simulink library "Garbage"  (R2023a)
settings: Solver=ode45  StartTime=0.0  StopTime=10.0  RelTol=1e-3  EnableMultiTasking=on
undefined symbols (not defined in the file or in its folder): c, ending_column_index, ending_row_index, framebuffer_16x1, framebuffer_2x2, framebuffer_4x4, framebuffer_8x8, HEIGHT, hot, POS_X, POS_Y, starting_column_index, starting_row_index, WIDTH
libraries: Memory_Blocks_Lib x459, simulink x235, simulink_extras x132, dspmtrx3 x116, Bit_Multiplexers_Lib x67, Bit_Demultiplexers_Lib x49, Byte_Multiplexers_Lib x40, Miscellaneous x38, Computer_Library x17, Decoders_Lib x14, commutil2 x12, Arithmetic_Operations_Lib x8
(part 14 of 26)

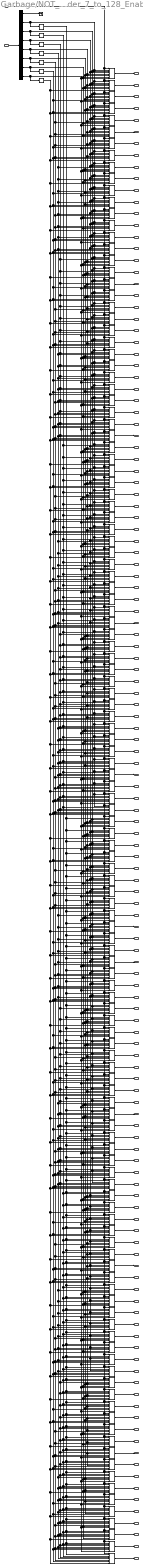
== system Garbage/NOT_USED_Decoder_7_to_128_Enable ==
  Enable(1b) : Inport
  Addr(1B) : Inport  Port=2
  Lo`gical Operator{1,3,5..6} (4 alike) : Logic  Operator=NOT  IconShape=distinctive  AllPortsSameDT=off  OutDataTypeStr=boolean
  Logical Operator{1..4,6..21,24..81,84..111,113..134} (128 alike) : Logic  Inputs=8  IconShape=distinctive  AllPortsSameDT=off  OutDataTypeStr=boolean
  Logical Operator112 : Logic  Operator=NOT  IconShape=distinctive  AllPortsSameDT=off  OutDataTypeStr=boolean
  Logical Operator82 : Logic  Operator=NOT  IconShape=distinctive  AllPortsSameDT=off  OutDataTypeStr=boolean
  Logical Operator83 : Logic  Operator=NOT  IconShape=distinctive  AllPortsSameDT=off  OutDataTypeStr=boolean
  Terminator : Terminator
  xyz1 : BusSelector  OutputSignals=signal1,signal2,signal3,signal4,signal5,signal6,signal7,signal8
  Select_127(1b) : Outport
  Select_126(1b) : Outport  Port=2
  Select_125(1b) : Outport  Port=3
  Select_124(1b) : Outport  Port=4
  Select_123(1b) : Outport  Port=5
  Select_122(1b) : Outport  Port=6
  Select_121(1b) : Outport  Port=7
  Select_120(1b) : Outport  Port=8
  Select_119(1b) : Outport  Port=9
  Select_118(1b) : Outport  Port=10
  Select_117(1b) : Outport  Port=11
  Select_116(1b) : Outport  Port=12
  Select_115(1b) : Outport  Port=13
  Select_114(1b) : Outport  Port=14
  Select_113(1b) : Outport  Port=15
  Select_112(1b) : Outport  Port=16
  Select_111(1b) : Outport  Port=17
  Select_110(1b) : Outport  Port=18
  Select_109(1b) : Outport  Port=19
  Select_108(1b) : Outport  Port=20
  Select_107(1b) : Outport  Port=21
  Select_106(1b) : Outport  Port=22
  Select_105(1b) : Outport  Port=23
  Select_104(1b) : Outport  Port=24
  Select_103(1b) : Outport  Port=25
  Select_102(1b) : Outport  Port=26
  Select_101(1b) : Outport  Port=27
  Select_100(1b) : Outport  Port=28
  Select_099(1b) : Outport  Port=29
  Select_098(1b) : Outport  Port=30
  Select_097(1b) : Outport  Port=31
  Select_096(1b) : Outport  Port=32
  Select_095(1b) : Outport  Port=33
  Select_094(1b) : Outport  Port=34
  Select_093(1b) : Outport  Port=35
  Select_092(1b) : Outport  Port=36
  Select_091(1b) : Outport  Port=37
  Select_090(1b) : Outport  Port=38
  Select_089(1b) : Outport  Port=39
  Select_088(1b) : Outport  Port=40
  Select_087(1b) : Outport  Port=41
  Select_086(1b) : Outport  Port=42
  Select_085(1b) : Outport  Port=43
  Select_084(1b) : Outport  Port=44
  Select_083(1b) : Outport  Port=45
  Select_082(1b) : Outport  Port=46
  Select_081(1b) : Outport  Port=47
  Select_080(1b) : Outport  Port=48
  Select_079(1b) : Outport  Port=49
  Select_078(1b) : Outport  Port=50
  Select_077(1b) : Outport  Port=51
  Select_076(1b) : Outport  Port=52
  Select_075(1b) : Outport  Port=53
  Select_074(1b) : Outport  Port=54
  Select_073(1b) : Outport  Port=55
  Select_072(1b) : Outport  Port=56
  Select_071(1b) : Outport  Port=57
  Select_070(1b) : Outport  Port=58
  Select_069(1b) : Outport  Port=59
  Select_068(1b) : Outport  Port=60
  Select_067(1b) : Outport  Port=61
  Select_066(1b) : Outport  Port=62
  Select_065(1b) : Outport  Port=63
  Select_064(1b) : Outport  Port=64
  Select_063(1b) : Outport  Port=65
  Select_062(1b) : Outport  Port=66
  Select_061(1b) : Outport  Port=67
  Select_060(1b) : Outport  Port=68
  Select_059(1b) : Outport  Port=69
  Select_058(1b) : Outport  Port=70
  Select_057(1b) : Outport  Port=71
  Select_056(1b) : Outport  Port=72
  Select_055(1b) : Outport  Port=73
  Select_054(1b) : Outport  Port=74
  Select_053(1b) : Outport  Port=75
  Select_052(1b) : Outport  Port=76
  Select_051(1b) : Outport  Port=77
  Select_050(1b) : Outport  Port=78
  Select_049(1b) : Outport  Port=79
  Select_048(1b) : Outport  Port=80
  Select_047(1b) : Outport  Port=81
  Select_046(1b) : Outport  Port=82
  Select_045(1b) : Outport  Port=83
  Select_044(1b) : Outport  Port=84
  Select_043(1b) : Outport  Port=85
  Select_042(1b) : Outport  Port=86
  Select_041(1b) : Outport  Port=87
  Select_040(1b) : Outport  Port=88
  Select_039(1b) : Outport  Port=89
  Select_038(1b) : Outport  Port=90
  Select_037(1b) : Outport  Port=91
  Select_036(1b) : Outport  Port=92
  Select_035(1b) : Outport  Port=93
  Select_034(1b) : Outport  Port=94
  Select_033(1b) : Outport  Port=95
  Select_032(1b) : Outport  Port=96
  Select_031(1b) : Outport  Port=97
  Select_030(1b) : Outport  Port=98
  Select_029(1b) : Outport  Port=99
  Select_028(1b) : Outport  Port=100
  Select_027(1b) : Outport  Port=101
  Select_026(1b) : Outport  Port=102
  Select_025(1b) : Outport  Port=103
  Select_024(1b) : Outport  Port=104
  Select_023(1b) : Outport  Port=105
  Select_022(1b) : Outport  Port=106
  Select_021(1b) : Outport  Port=107
  Select_020(1b) : Outport  Port=108
  Select_019(1b) : Outport  Port=109
  Select_018(1b) : Outport  Port=110
  Select_017(1b) : Outport  Port=111
  Select_016(1b) : Outport  Port=112
  Select_015(1b) : Outport  Port=113
  Select_014(1b) : Outport  Port=114
  Select_013(1b) : Outport  Port=115
  Select_012(1b) : Outport  Port=116
  Select_011(1b) : Outport  Port=117
  Select_010(1b) : Outport  Port=118
  Select_009(1b) : Outport  Port=119
  Select_008(1b) : Outport  Port=120
  Select_007(1b) : Outport  Port=121
  Select_006(1b) : Outport  Port=122
  Select_005(1b) : Outport  Port=123
  Select_004(1b) : Outport  Port=124
  Select_003(1b) : Outport  Port=125
  Select_002(1b) : Outport  Port=126
  Select_001(1b) : Outport  Port=127
  Select_000(1b) : Outport  Port=128
  wires:
  Logical Operator16/1 -> Select_048(1b)/1
  Logical Operator15/1 -> Select_049(1b)/1
  Logical Operator14/1 -> Select_050(1b)/1
  Logical Operator13/1 -> Select_051(1b)/1
  Logical Operator12/1 -> Select_052(1b)/1
  Logical Operator11/1 -> Select_053(1b)/1
  Logical Operator10/1 -> Select_054(1b)/1
  Logical Operator9/1 -> Select_055(1b)/1
  Logical Operator8/1 -> Select_056(1b)/1
  Logical Operator7/1 -> Select_057(1b)/1
  Logical Operator6/1 -> Select_058(1b)/1
  Logical Operator4/1 -> Select_059(1b)/1
  Logical Operator1/1 -> Select_062(1b)/1
  Logical Operator2/1 -> Select_061(1b)/1
  Logical Operator3/1 -> Select_060(1b)/1
  Logical Operator63/1 -> Select_063(1b)/1
  Logical Operator26/1 -> Select_032(1b)/1
  Logical Operator25/1 -> Select_033(1b)/1
  Logical Operator24/1 -> Select_034(1b)/1
  Logical Operator21/1 -> Select_035(1b)/1
  Logical Operator20/1 -> Select_036(1b)/1
  Logical Operator19/1 -> Select_037(1b)/1
  Logical Operator18/1 -> Select_038(1b)/1
  Logical Operator34/1 -> Select_039(1b)/1
  Logical Operator33/1 -> Select_040(1b)/1
  Logical Operator32/1 -> Select_041(1b)/1
  Logical Operator30/1 -> Select_042(1b)/1
  Logical Operator29/1 -> Select_043(1b)/1
  Logical Operator17/1 -> Select_046(1b)/1
  Logical Operator27/1 -> Select_045(1b)/1
  Logical Operator28/1 -> Select_044(1b)/1
  Logical Operator31/1 -> Select_047(1b)/1
  xyz1/3 -> Logical Operator76/3, Logical Operator17/3, Logical Operator26/3, Logical Operator25/3, Logical Operator24/3, Logical Operator21/3, Logical Operator20/3, Logical Operator19/3, Logical Operator18/3, Logical Operator34/3, Logical Operator33/3, Logical Operator32/3, Logical Operator30/3, Logical Operator29/3, Logical Operator28/3, Logical Operator27/3, Logical Operator31/3, Logical Operator16/3, Logical Operator15/3, Logical Operator14/3, Logical Operator13/3, Logical Operator12/3, Logical Operator11/3, Logical Operator10/3, Logical Operator9/3, Logical Operator8/3, Logical Operator7/3, Logical Operator6/3, Logical Operator4/3, Logical Operator3/3, Logical Operator2/3, Logical Operator1/3, Logical Operator63/3, Logical Operator86/3, Logical Operator85/3, Logical Operator84/3, Logical Operator81/3, Logical Operator80/3, Logical Operator78/3, Logical Operator77/3, Logical Operator95/3, Logical Operator94/3, Logical Operator93/3, Logical Operator91/3, Logical Operator89/3, Logical Operator88/3, Logical Operator87/3, Logical Operator92/3, Logical Operator75/3, Logical Operator74/3, Logical Operator73/3, Logical Operator72/3, Logical Operator71/3, Logical Operator70/3, Logical Operator69/3, Logical Operator134/3, Logical Operator133/3, Logical Operator132/3, Logical Operator123/3, Logical Operator101/3, Logical Operator90/3, Logical Operator79/3, Logical Operator68/3, Logical Operator127/3, Lo`gical Operator5/1  "<signal3>"
  Logical Operator42/1 -> Select_016(1b)/1
  Logical Operator41/1 -> Select_017(1b)/1
  Logical Operator40/1 -> Select_018(1b)/1
  Logical Operator39/1 -> Select_019(1b)/1
  Logical Operator38/1 -> Select_020(1b)/1
  Logical Operator37/1 -> Select_021(1b)/1
  Logical Operator36/1 -> Select_022(1b)/1
  Logical Operator50/1 -> Select_023(1b)/1
  Logical Operator49/1 -> Select_024(1b)/1
  Logical Operator48/1 -> Select_025(1b)/1
  Logical Operator46/1 -> Select_026(1b)/1
  Logical Operator45/1 -> Select_027(1b)/1
  Logical Operator35/1 -> Select_030(1b)/1
  Logical Operator43/1 -> Select_029(1b)/1
  Logical Operator44/1 -> Select_028(1b)/1
  Logical Operator47/1 -> Select_031(1b)/1
  Logical Operator82/1 -> Logical Operator93/7, Logical Operator105/7, Logical Operator106/7, Logical Operator122/7, Logical Operator124/7, Logical Operator32/7, Logical Operator43/7, Logical Operator44/7, Logical Operator59/7, Logical Operator60/7, Logical Operator58/7, Logical Operator57/7, Logical Operator54/7, Logical Operator53/7, Logical Operator66/7, Logical Operator65/7, Logical Operator42/7, Logical Operator41/7, Logical Operator38/7, Logical Operator37/7, Logical Operator49/7, Logical Operator48/7, Logical Operator26/7, Logical Operator25/7, Logical Operator20/7, Logical Operator19/7, Logical Operator33/7, Logical Operator28/7, Logical Operator27/7, Logical Operator16/7, Logical Operator15/7, Logical Operator12/7, Logical Operator11/7, Logical Operator8/7, Logical Operator7/7, Logical Operator3/7, Logical Operator2/7, Logical Operator121/7, Logical Operator120/7, Logical Operator117/7, Logical Operator116/7, Logical Operator130/7, Logical Operator129/7, Logical Operator104/7, Logical Operator103/7, Logical Operator99/7, Logical Operator98/7, Logical Operator111/7, Logical Operator110/7, Logical Operator86/7, Logical Operator85/7, Logical Operator80/7, Logical Operator78/7, Logical Operator94/7, Logical Operator88/7, Logical Operator87/7, Logical Operator75/7, Logical Operator74/7, Logical Operator71/7, Logical Operator70/7, Logical Operator133/7, Logical Operator132/7, Logical Operator90/7, Logical Operator79/7
  xyz1/8 -> Logical Operator83/1, Logical Operator127/8, Logical Operator63/8, Logical Operator57/8, Logical Operator55/8, Logical Operator53/8, Logical Operator67/8, Logical Operator65/8, Logical Operator61/8, Logical Operator59/8, Logical Operator64/8, Logical Operator41/8, Logical Operator39/8, Logical Operator37/8, Logical Operator50/8, Logical Operator48/8, Logical Operator45/8, Logical Operator43/8, Logical Operator47/8, Logical Operator25/8, Logical Operator21/8, Logical Operator19/8, Logical Operator34/8, Logical Operator32/8, Logical Operator29/8, Logical Operator27/8, Logical Operator31/8, Logical Operator15/8, Logical Operator13/8, Logical Operator11/8, Logical Operator9/8, Logical Operator7/8, Logical Operator4/8, Logical Operator2/8, Logical Operator120/8, Logical Operator118/8, Logical Operator116/8, Logical Operator131/8, Logical Operator129/8, Logical Operator125/8, Logical Operator122/8, Logical Operator128/8, Logical Operator103/8, Logical Operator100/8, Logical Operator98/8, Logical Operator113/8, Logical Operator110/8, Logical Operator107/8, Logical Operator105/8, Logical Operator109/8, Logical Operator85/8, Logical Operator81/8, Logical Operator78/8, Logical Operator95/8, Logical Operator93/8, Logical Operator89/8, Logical Operator87/8, Logical Operator92/8, Logical Operator74/8, Logical Operator72/8, Logical Operator70/8, Logical Operator134/8, Logical Operator132/8, Logical Operator101/8, Logical Operator79/8  "<signal8>"
  Logical Operator83/1 -> Logical Operator86/8, Logical Operator104/8, Logical Operator26/8, Logical Operator42/8, Logical Operator58/8, Logical Operator56/8, Logical Operator54/8, Logical Operator52/8, Logical Operator66/8, Logical Operator62/8, Logical Operator60/8, Logical Operator51/8, Logical Operator40/8, Logical Operator38/8, Logical Operator36/8, Logical Operator49/8, Logical Operator46/8, Logical Operator44/8, Logical Operator35/8, Logical Operator24/8, Logical Operator20/8, Logical Operator18/8, Logical Operator33/8, Logical Operator30/8, Logical Operator28/8, Logical Operator17/8, Logical Operator16/8, Logical Operator14/8, Logical Operator12/8, Logical Operator10/8, Logical Operator8/8, Logical Operator6/8, Logical Operator3/8, Logical Operator1/8, Logical Operator121/8, Logical Operator119/8, Logical Operator117/8, Logical Operator115/8, Logical Operator130/8, Logical Operator126/8, Logical Operator124/8, Logical Operator114/8, Logical Operator102/8, Logical Operator99/8, Logical Operator97/8, Logical Operator111/8, Logical Operator108/8, Logical Operator106/8, Logical Operator96/8, Logical Operator84/8, Logical Operator80/8, Logical Operator77/8, Logical Operator94/8, Logical Operator91/8, Logical Operator88/8, Logical Operator76/8, Logical Operator75/8, Logical Operator73/8, Logical Operator71/8, Logical Operator69/8, Logical Operator133/8, Logical Operator123/8, Logical Operator90/8, Logical Operator68/8
  Logical Operator112/1 -> Logical Operator86/6, Logical Operator26/6, Logical Operator58/6, Logical Operator57/6, Logical Operator56/6, Logical Operator55/6, Logical Operator66/6, Logical Operator65/6, Logical Operator62/6, Logical Operator61/6, Logical Operator42/6, Logical Operator41/6, Logical Operator40/6, Logical Operator39/6, Logical Operator49/6, Logical Operator48/6, Logical Operator46/6, Logical Operator45/6, Logical Operator25/6, Logical Operator24/6, Logical Operator21/6, Logical Operator33/6, Logical Operator32/6, Logical Operator30/6, Logical Operator29/6, Logical Operator16/6, Logical Operator15/6, Logical Operator14/6, Logical Operator13/6, Logical Operator8/6, Logical Operator7/6, Logical Operator6/6, Logical Operator4/6, Logical Operator121/6, Logical Operator120/6, Logical Operator119/6, Logical Operator118/6, Logical Operator130/6, Logical Operator129/6, Logical Operator126/6, Logical Operator125/6, Logical Operator104/6, Logical Operator103/6, Logical Operator102/6, Logical Operator100/6, Logical Operator111/6, Logical Operator110/6, Logical Operator108/6, Logical Operator107/6, Logical Operator85/6, Logical Operator84/6, Logical Operator81/6, Logical Operator94/6, Logical Operator93/6, Logical Operator91/6, Logical Operator89/6, Logical Operator75/6, Logical Operator74/6, Logical Operator73/6, Logical Operator72/6, Logical Operator133/6, Logical Operator132/6, Logical Operator123/6, Logical Operator101/6
  xyz1/5 -> Lo`gical Operator6/1, Logical Operator127/5, Logical Operator68/5, Logical Operator79/5, Logical Operator90/5, Logical Operator101/5, Logical Operator123/5, Logical Operator132/5, Logical Operator133/5, Logical Operator92/5, Logical Operator76/5, Logical Operator87/5, Logical Operator88/5, Logical Operator89/5, Logical Operator91/5, Logical Operator94/5, Logical Operator109/5, Logical Operator96/5, Logical Operator105/5, Logical Operator106/5, Logical Operator107/5, Logical Operator108/5, Logical Operator110/5, Logical Operator111/5, Logical Operator128/5, Logical Operator114/5, Logical Operator122/5, Logical Operator124/5, Logical Operator125/5, Logical Operator126/5, Logical Operator63/5, Logical Operator1/5, Logical Operator2/5, Logical Operator3/5, Logical Operator4/5, Logical Operator6/5, Logical Operator7/5, Logical Operator8/5, Logical Operator31/5, Logical Operator17/5, Logical Operator27/5, Logical Operator28/5, Logical Operator29/5, Logical Operator30/5, Logical Operator33/5, Logical Operator47/5, Logical Operator35/5, Logical Operator43/5, Logical Operator44/5, Logical Operator45/5, Logical Operator46/5, Logical Operator48/5, Logical Operator49/5, Logical Operator64/5, Logical Operator51/5, Logical Operator59/5, Logical Operator60/5, Logical Operator61/5, Logical Operator62/5, Logical Operator66/5, Logical Operator65/5, Logical Operator32/5, Logical Operator130/5, Logical Operator129/5, Logical Operator93/5  "<signal5>"
  xyz1/7 -> Logical Operator82/1, Logical Operator127/7, Logical Operator91/7, Logical Operator95/7, Logical Operator84/7, Logical Operator109/7, Logical Operator119/7, Logical Operator63/7, Logical Operator30/7, Logical Operator34/7, Logical Operator24/7, Logical Operator47/7, Logical Operator56/7, Logical Operator55/7, Logical Operator52/7, Logical Operator67/7, Logical Operator62/7, Logical Operator61/7, Logical Operator51/7, Logical Operator64/7, Logical Operator40/7, Logical Operator39/7, Logical Operator36/7, Logical Operator50/7, Logical Operator46/7, Logical Operator45/7, Logical Operator35/7, Logical Operator21/7, Logical Operator18/7, Logical Operator29/7, Logical Operator17/7, Logical Operator31/7, Logical Operator14/7, Logical Operator13/7, Logical Operator10/7, Logical Operator9/7, Logical Operator6/7, Logical Operator4/7, Logical Operator1/7, Logical Operator118/7, Logical Operator115/7, Logical Operator131/7, Logical Operator126/7, Logical Operator125/7, Logical Operator114/7, Logical Operator128/7, Logical Operator102/7, Logical Operator100/7, Logical Operator97/7, Logical Operator113/7, Logical Operator108/7, Logical Operator107/7, Logical Operator96/7, Logical Operator81/7, Logical Operator77/7, Logical Operator89/7, Logical Operator76/7, Logical Operator92/7, Logical Operator73/7, Logical Operator72/7, Logical Operator69/7, Logical Operator134/7, Logical Operator123/7, Logical Operator101/7, Logical Operator68/7  "<signal7>"
  xyz1/6 -> Logical Operator112/1, Logical Operator127/6, Logical Operator68/6, Logical Operator134/6, Logical Operator69/6, Logical Operator70/6, Logical Operator71/6, Logical Operator92/6, Logical Operator76/6, Logical Operator95/6, Logical Operator77/6, Logical Operator78/6, Logical Operator80/6, Logical Operator109/6, Logical Operator96/6, Logical Operator113/6, Logical Operator97/6, Logical Operator98/6, Logical Operator99/6, Logical Operator128/6, Logical Operator114/6, Logical Operator131/6, Logical Operator115/6, Logical Operator63/6, Logical Operator1/6, Logical Operator9/6, Logical Operator10/6, Logical Operator11/6, Logical Operator12/6, Logical Operator31/6, Logical Operator17/6, Logical Operator34/6, Logical Operator18/6, Logical Operator19/6, Logical Operator20/6, Logical Operator47/6, Logical Operator35/6, Logical Operator50/6, Logical Operator36/6, Logical Operator37/6, Logical Operator38/6, Logical Operator64/6, Logical Operator51/6, Logical Operator67/6, Logical Operator52/6, Logical Operator54/6, Logical Operator53/6, Logical Operator60/6, Logical Operator59/6, Logical Operator44/6, Logical Operator43/6, Logical Operator28/6, Logical Operator27/6, Logical Operator3/6, Logical Operator2/6, Logical Operator117/6, Logical Operator116/6, Logical Operator124/6, Logical Operator122/6, Logical Operator106/6, Logical Operator105/6, Logical Operator88/6, Logical Operator87/6, Logical Operator90/6, Logical Operator79/6  "<signal6>"
  Lo`gical Operator6/1 -> Logical Operator77/5, Logical Operator78/5, Logical Operator80/5, Logical Operator81/5, Logical Operator84/5, Logical Operator85/5, Logical Operator113/5, Logical Operator97/5, Logical Operator98/5, Logical Operator99/5, Logical Operator100/5, Logical Operator102/5, Logical Operator103/5, Logical Operator131/5, Logical Operator115/5, Logical Operator116/5, Logical Operator117/5, Logical Operator118/5, Logical Operator119/5, Logical Operator18/5, Logical Operator19/5, Logical Operator20/5, Logical Operator21/5, Logical Operator24/5, Logical Operator25/5, Logical Operator50/5, Logical Operator36/5, Logical Operator37/5, Logical Operator38/5, Logical Operator39/5, Logical Operator40/5, Logical Operator41/5, Logical Operator67/5, Logical Operator52/5, Logical Operator53/5, Logical Operator54/5, Logical Operator55/5, Logical Operator56/5, Logical Operator58/5, Logical Operator57/5, Logical Operator42/5, Logical Operator26/5, Logical Operator34/5, Logical Operator16/5, Logical Operator15/5, Logical Operator14/5, Logical Operator13/5, Logical Operator12/5, Logical Operator11/5, Logical Operator10/5, Logical Operator9/5, Logical Operator121/5, Logical Operator120/5, Logical Operator104/5, Logical Operator86/5, Logical Operator95/5, Logical Operator75/5, Logical Operator74/5, Logical Operator73/5, Logical Operator72/5, Logical Operator71/5, Logical Operator70/5, Logical Operator69/5, Logical Operator134/5
  Logical Operator58/1 -> Select_000(1b)/1
  Logical Operator57/1 -> Select_001(1b)/1
  Logical Operator56/1 -> Select_002(1b)/1
  Logical Operator55/1 -> Select_003(1b)/1
  Logical Operator54/1 -> Select_004(1b)/1
  Logical Operator53/1 -> Select_005(1b)/1
  Logical Operator52/1 -> Select_006(1b)/1
  Logical Operator67/1 -> Select_007(1b)/1
  Logical Operator66/1 -> Select_008(1b)/1
  Logical Operator65/1 -> Select_009(1b)/1
  Logical Operator62/1 -> Select_010(1b)/1
  Logical Operator61/1 -> Select_011(1b)/1
  Logical Operator51/1 -> Select_014(1b)/1
  Logical Operator59/1 -> Select_013(1b)/1
  Logical Operator60/1 -> Select_012(1b)/1
  Logical Operator64/1 -> Select_015(1b)/1
  Lo`gical Operator3/1 -> Logical Operator78/4, Logical Operator86/4, Logical Operator122/4, Logical Operator126/4, Logical Operator19/4, Logical Operator26/4, Logical Operator59/4, Logical Operator62/4, Logical Operator58/4, Logical Operator57/4, Logical Operator56/4, Logical Operator55/4, Logical Operator54/4, Logical Operator53/4, Logical Operator52/4, Logical Operator67/4, Logical Operator66/4, Logical Operator65/4, Logical Operator61/4, Logical Operator60/4, Logical Operator51/4, Logical Operator64/4, Logical Operator25/4, Logical Operator24/4, Logical Operator21/4, Logical Operator20/4, Logical Operator18/4, Logical Operator34/4, Logical Operator33/4, Logical Operator32/4, Logical Operator30/4, Logical Operator29/4, Logical Operator28/4, Logical Operator27/4, Logical Operator17/4, Logical Operator31/4, Logical Operator121/4, Logical Operator120/4, Logical Operator119/4, Logical Operator118/4, Logical Operator117/4, Logical Operator116/4, Logical Operator115/4, Logical Operator131/4, Logical Operator130/4, Logical Operator129/4, Logical Operator125/4, Logical Operator124/4, Logical Operator114/4, Logical Operator128/4, Logical Operator85/4, Logical Operator84/4, Logical Operator81/4, Logical Operator80/4, Logical Operator77/4, Logical Operator95/4, Logical Operator94/4, Logical Operator93/4, Logical Operator91/4, Logical Operator89/4, Logical Operator88/4, Logical Operator87/4, Logical Operator76/4, Logical Operator92/4
  xyz1/4 -> Lo`gical Operator3/1, Logical Operator109/4, Logical Operator106/4, Logical Operator107/4, Logical Operator108/4, Logical Operator111/4, Logical Operator113/4, Logical Operator98/4, Logical Operator100/4, Logical Operator47/4, Logical Operator44/4, Logical Operator45/4, Logical Operator46/4, Logical Operator49/4, Logical Operator50/4, Logical Operator37/4, Logical Operator39/4, Logical Operator42/4, Logical Operator41/4, Logical Operator40/4, Logical Operator38/4, Logical Operator36/4, Logical Operator48/4, Logical Operator43/4, Logical Operator35/4, Logical Operator16/4, Logical Operator15/4, Logical Operator14/4, Logical Operator13/4, Logical Operator12/4, Logical Operator11/4, Logical Operator10/4, Logical Operator9/4, Logical Operator8/4, Logical Operator7/4, Logical Operator6/4, Logical Operator4/4, Logical Operator3/4, Logical Operator2/4, Logical Operator1/4, Logical Operator63/4, Logical Operator104/4, Logical Operator103/4, Logical Operator102/4, Logical Operator99/4, Logical Operator97/4, Logical Operator110/4, Logical Operator105/4, Logical Operator96/4, Logical Operator75/4, Logical Operator74/4, Logical Operator73/4, Logical Operator72/4, Logical Operator71/4, Logical Operator70/4, Logical Operator69/4, Logical Operator134/4, Logical Operator133/4, Logical Operator132/4, Logical Operator123/4, Logical Operator101/4, Logical Operator90/4, Logical Operator79/4, Logical Operator68/4, Logical Operator127/4  "<signal4>"
  Lo`gical Operator5/1 -> Logical Operator107/3, Logical Operator125/3, Logical Operator126/3, Logical Operator129/3, Logical Operator130/3, Logical Operator131/3, Logical Operator115/3, Logical Operator116/3, Logical Operator117/3, Logical Operator118/3, Logical Operator119/3, Logical Operator45/3, Logical Operator61/3, Logical Operator62/3, Logical Operator65/3, Logical Operator66/3, Logical Operator67/3, Logical Operator52/3, Logical Operator53/3, Logical Operator54/3, Logical Operator55/3, Logical Operator56/3, Logical Operator58/3, Logical Operator57/3, Logical Operator60/3, Logical Operator59/3, Logical Operator51/3, Logical Operator64/3, Logical Operator42/3, Logical Operator41/3, Logical Operator40/3, Logical Operator39/3, Logical Operator38/3, Logical Operator37/3, Logical Operator36/3, Logical Operator50/3, Logical Operator49/3, Logical Operator48/3, Logical Operator46/3, Logical Operator44/3, Logical Operator43/3, Logical Operator35/3, Logical Operator47/3, Logical Operator121/3, Logical Operator120/3, Logical Operator124/3, Logical Operator122/3, Logical Operator114/3, Logical Operator128/3, Logical Operator104/3, Logical Operator103/3, Logical Operator102/3, Logical Operator100/3, Logical Operator99/3, Logical Operator98/3, Logical Operator97/3, Logical Operator113/3, Logical Operator111/3, Logical Operator110/3, Logical Operator108/3, Logical Operator106/3, Logical Operator105/3, Logical Operator96/3, Logical Operator109/3
  Enable(1b)/1 -> Logical Operator124/1, Logical Operator125/1, Logical Operator60/1, Logical Operator61/1, Logical Operator58/1, Logical Operator57/1, Logical Operator56/1, Logical Operator55/1, Logical Operator54/1, Logical Operator53/1, Logical Operator52/1, Logical Operator67/1, Logical Operator66/1, Logical Operator65/1, Logical Operator62/1, Logical Operator59/1, Logical Operator51/1, Logical Operator64/1, Logical Operator42/1, Logical Operator41/1, Logical Operator40/1, Logical Operator39/1, Logical Operator38/1, Logical Operator37/1, Logical Operator36/1, Logical Operator50/1, Logical Operator49/1, Logical Operator48/1, Logical Operator46/1, Logical Operator45/1, Logical Operator44/1, Logical Operator43/1, Logical Operator35/1, Logical Operator47/1, Logical Operator26/1, Logical Operator25/1, Logical Operator24/1, Logical Operator21/1, Logical Operator20/1, Logical Operator19/1, Logical Operator18/1, Logical Operator34/1, Logical Operator33/1, Logical Operator32/1, Logical Operator30/1, Logical Operator29/1, Logical Operator28/1, Logical Operator27/1, Logical Operator17/1, Logical Operator31/1, Logical Operator16/1, Logical Operator15/1, Logical Operator14/1, Logical Operator13/1, Logical Operator12/1, Logical Operator11/1, Logical Operator10/1, Logical Operator9/1, Logical Operator8/1, Logical Operator7/1, Logical Operator6/1, Logical Operator4/1, Logical Operator3/1, Logical Operator2/1, Logical Operator1/1, Logical Operator63/1, Logical Operator121/1, Logical Operator120/1, Logical Operator119/1, Logical Operator118/1, Logical Operator117/1, Logical Operator116/1, Logical Operator115/1, Logical Operator131/1, Logical Operator130/1, Logical Operator129/1, Logical Operator126/1, Logical Operator122/1, Logical Operator114/1, Logical Operator128/1, Logical Operator104/1, Logical Operator103/1, Logical Operator102/1, Logical Operator100/1, Logical Operator99/1, Logical Operator98/1, Logical Operator97/1, Logical Operator113/1, Logical Operator111/1, Logical Operator110/1, Logical Operator108/1, Logical Operator107/1, Logical Operator106/1, Logical Operator105/1, Logical Operator96/1, Logical Operator109/1, Logical Operator86/1, Logical Operator85/1, Logical Operator84/1, Logical Operator81/1, Logical Operator80/1, Logical Operator78/1, Logical Operator77/1, Logical Operator95/1, Logical Operator94/1, Logical Operator93/1, Logical Operator91/1, Logical Operator89/1, Logical Operator88/1, Logical Operator87/1, Logical Operator76/1, Logical Operator92/1, Logical Operator75/1, Logical Operator74/1, Logical Operator73/1, Logical Operator72/1, Logical Operator71/1, Logical Operator70/1, Logical Operator69/1, Logical Operator134/1, Logical Operator133/1, Logical Operator132/1, Logical Operator123/1, Logical Operator101/1, Logical Operator90/1, Logical Operator79/1, Logical Operator68/1, Logical Operator127/1
  xyz1/2 -> Lo`gical Operator1/1, Logical Operator98/2, Logical Operator121/2, Logical Operator120/2, Logical Operator119/2, Logical Operator118/2, Logical Operator117/2, Logical Operator116/2, Logical Operator115/2, Logical Operator131/2, Logical Operator130/2, Logical Operator129/2, Logical Operator126/2, Logical Operator125/2, Logical Operator124/2, Logical Operator122/2, Logical Operator114/2, Logical Operator128/2, Logical Operator104/2, Logical Operator103/2, Logical Operator102/2, Logical Operator100/2, Logical Operator99/2, Logical Operator97/2, Logical Operator113/2, Logical Operator111/2, Logical Operator110/2, Logical Operator108/2, Logical Operator107/2, Logical Operator106/2, Logical Operator105/2, Logical Operator96/2, Logical Operator109/2, Logical Operator86/2, Logical Operator85/2, Logical Operator84/2, Logical Operator81/2, Logical Operator80/2, Logical Operator78/2, Logical Operator77/2, Logical Operator95/2, Logical Operator94/2, Logical Operator93/2, Logical Operator91/2, Logical Operator89/2, Logical Operator88/2, Logical Operator87/2, Logical Operator76/2, Logical Operator92/2, Logical Operator75/2, Logical Operator74/2, Logical Operator73/2, Logical Operator72/2, Logical Operator71/2, Logical Operator70/2, Logical Operator69/2, Logical Operator134/2, Logical Operator133/2, Logical Operator132/2, Logical Operator123/2, Logical Operator101/2, Logical Operator90/2, Logical Operator79/2, Logical Operator68/2, Logical Operator127/2  "<signal2>"
  Logical Operator75/1 -> Select_112(1b)/1
  Logical Operator74/1 -> Select_113(1b)/1
  Logical Operator73/1 -> Select_114(1b)/1
  Logical Operator72/1 -> Select_115(1b)/1
  Logical Operator71/1 -> Select_116(1b)/1
  Logical Operator70/1 -> Select_117(1b)/1
  Logical Operator69/1 -> Select_118(1b)/1
  Logical Operator134/1 -> Select_119(1b)/1
  Logical Operator133/1 -> Select_120(1b)/1
  Logical Operator132/1 -> Select_121(1b)/1
  Logical Operator123/1 -> Select_122(1b)/1
  Logical Operator101/1 -> Select_123(1b)/1
  Logical Operator68/1 -> Select_126(1b)/1
  Logical Operator79/1 -> Select_125(1b)/1
  Logical Operator90/1 -> Select_124(1b)/1
  Logical Operator127/1 -> Select_127(1b)/1
  Logical Operator86/1 -> Select_096(1b)/1
  Logical Operator85/1 -> Select_097(1b)/1
  Logical Operator84/1 -> Select_098(1b)/1
  Logical Operator81/1 -> Select_099(1b)/1
  Logical Operator80/1 -> Select_100(1b)/1
  Logical Operator78/1 -> Select_101(1b)/1
  Logical Operator77/1 -> Select_102(1b)/1
  Logical Operator95/1 -> Select_103(1b)/1
  Logical Operator94/1 -> Select_104(1b)/1
  Logical Operator93/1 -> Select_105(1b)/1
  Logical Operator91/1 -> Select_106(1b)/1
  Logical Operator89/1 -> Select_107(1b)/1
  Logical Operator76/1 -> Select_110(1b)/1
  Logical Operator87/1 -> Select_109(1b)/1
  Logical Operator88/1 -> Select_108(1b)/1
  Logical Operator92/1 -> Select_111(1b)/1
  Logical Operator104/1 -> Select_080(1b)/1
  Logical Operator103/1 -> Select_081(1b)/1
  Logical Operator102/1 -> Select_082(1b)/1
  Logical Operator100/1 -> Select_083(1b)/1
  Logical Operator99/1 -> Select_084(1b)/1
  Logical Operator98/1 -> Select_085(1b)/1
  Logical Operator97/1 -> Select_086(1b)/1
  Logical Operator113/1 -> Select_087(1b)/1
  Logical Operator111/1 -> Select_088(1b)/1
  Logical Operator110/1 -> Select_089(1b)/1
  Logical Operator108/1 -> Select_090(1b)/1
  Logical Operator107/1 -> Select_091(1b)/1
  Logical Operator96/1 -> Select_094(1b)/1
  Logical Operator105/1 -> Select_093(1b)/1
  Logical Operator106/1 -> Select_092(1b)/1
  Logical Operator109/1 -> Select_095(1b)/1
  Logical Operator121/1 -> Select_064(1b)/1
  Logical Operator120/1 -> Select_065(1b)/1
  Logical Operator119/1 -> Select_066(1b)/1
  Logical Operator118/1 -> Select_067(1b)/1
  Logical Operator117/1 -> Select_068(1b)/1
  Logical Operator116/1 -> Select_069(1b)/1
  Logical Operator115/1 -> Select_070(1b)/1
  Logical Operator131/1 -> Select_071(1b)/1
  Logical Operator130/1 -> Select_072(1b)/1
  Logical Operator129/1 -> Select_073(1b)/1
  Logical Operator126/1 -> Select_074(1b)/1
  Logical Operator125/1 -> Select_075(1b)/1
  Logical Operator114/1 -> Select_078(1b)/1
  Logical Operator122/1 -> Select_077(1b)/1
  Logical Operator124/1 -> Select_076(1b)/1
  Logical Operator128/1 -> Select_079(1b)/1
  Lo`gical Operator1/1 -> Logical Operator63/2, Logical Operator1/2, Logical Operator2/2, Logical Operator3/2, Logical Operator4/2, Logical Operator6/2, Logical Operator7/2, Logical Operator8/2, Logical Operator9/2, Logical Operator10/2, Logical Operator11/2, Logical Operator12/2, Logical Operator13/2, Logical Operator14/2, Logical Operator15/2, Logical Operator16/2, Logical Operator31/2, Logical Operator17/2, Logical Operator27/2, Logical Operator28/2, Logical Operator29/2, Logical Operator30/2, Logical Operator32/2, Logical Operator33/2, Logical Operator34/2, Logical Operator18/2, Logical Operator19/2, Logical Operator20/2, Logical Operator21/2, Logical Operator24/2, Logical Operator25/2, Logical Operator26/2, Logical Operator47/2, Logical Operator35/2, Logical Operator43/2, Logical Operator44/2, Logical Operator45/2, Logical Operator46/2, Logical Operator48/2, Logical Operator49/2, Logical Operator50/2, Logical Operator36/2, Logical Operator37/2, Logical Operator38/2, Logical Operator39/2, Logical Operator40/2, Logical Operator41/2, Logical Operator42/2, Logical Operator64/2, Logical Operator51/2, Logical Operator59/2, Logical Operator60/2, Logical Operator61/2, Logical Operator62/2, Logical Operator65/2, Logical Operator66/2, Logical Operator67/2, Logical Operator52/2, Logical Operator53/2, Logical Operator54/2, Logical Operator55/2, Logical Operator56/2, Logical Operator57/2, Logical Operator58/2
  Addr(1B)/1 -> xyz1/1
  xyz1/1 -> Terminator/1  "<signal1>"
